simulink model "ClimaCmftStMgr"  (R2022b)
settings: Solver=FixedStepDiscrete  StartTime=0.0  StopTime=10.0  FixedStep=0.1  RelTol=1e-3
(part 1 of 2)
settings Model: Creator=blue.ge  LastModifiedBy=blue.ge
settings DataIOCC: SaveFormat=StructureWithTime
settings OptimizationCC: DefaultParameterBehavior=Inlined  EnhancedBackFolding=on  PassReuseOutputArgsAs=Individual arguments  ZeroExternalMemoryAtStartup=off  ZeroInternalMemoryAtStartup=off  OptimizeBlockOrder=speed  DifferentSizesBufferReuse=on  OptimizationCustomize=off  EfficientTunableParamExpr=on
settings DebuggingCC: ParameterTunabilityLossMsg=error
settings ModelReferenceCC: ModelReferenceNumInstancesAllowed=Single
settings SFSimCC: MATLABDynamicMemAlloc=off
settings RTWCC: Description=AUTOSAR  SystemTargetFile=autosar.tlc  GenCodeOnly=on  TemplateMakefile=ert_default_tmf
settings CodeAppCC: ForceParamTrailComments=on  IgnoreCustomStorageClasses=off  OperatorAnnotations=on  SimulinkDataObjDesc=on  SFDataObjDesc=on  InsertBlockDesc=on
settings STFCustomTargetCC: TargetLangStandard=C99 (ISO)  MultiwordLength=256  ParMdlRefBuildCompliant=on  CompOptLevelCompliant=on  IncludeMdlTerminateFcn=off  CombineOutputUpdateFcns=on  SuppressErrorStatus=on  ERTCustomFileBanners=on  SupportNonFinite=off  SupportComplex=off  SupportContinuousTime=off  SupportNonInlinedSFcns=off  RemoveResetFunc=on  MATLABClassNameForMDSCustomization=Simulink.SoftwareTarget.ERTCustomization  AutosarCompliant=on  UseToolchainInfoCompliant=on  LookupTableObjectStructAxisOrder=2,1,3,4,...  LUTObjectStructOrderEvenSpacing=Size,Table,Breakpoints  SystemTargetFile=autosar.tlc
settings ERTTargetCC: TargetLangStandard=C99 (ISO)  ConcurrentExecutionCompliant=off  IncludeMdlTerminateFcn=off  SuppressErrorStatus=on  SupportNonFinite=off  SupportComplex=off  ModelStepFunctionPrototypeControlCompliant=off  CPPClassGenCompliant=off  AutosarCompliant=on  LookupTableObjectStructAxisOrder=2,1,3,4,...  LUTObjectStructOrderEvenSpacing=Size,Table,Breakpoints  InlinedParameterPlacement=NonHierarchical  ERTCustomFileTemplate=example_file_process.tlc

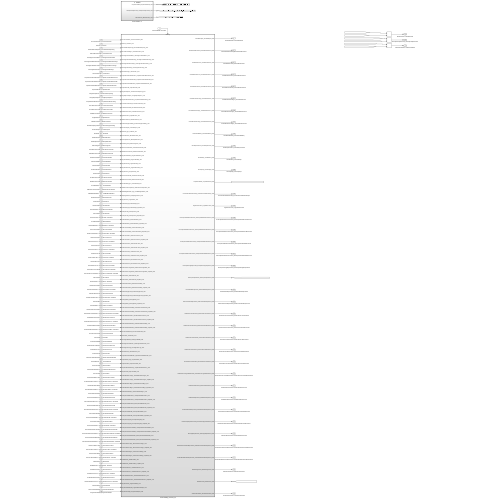
[thumbnail: Simulink's stored preview of the top-level diagram]
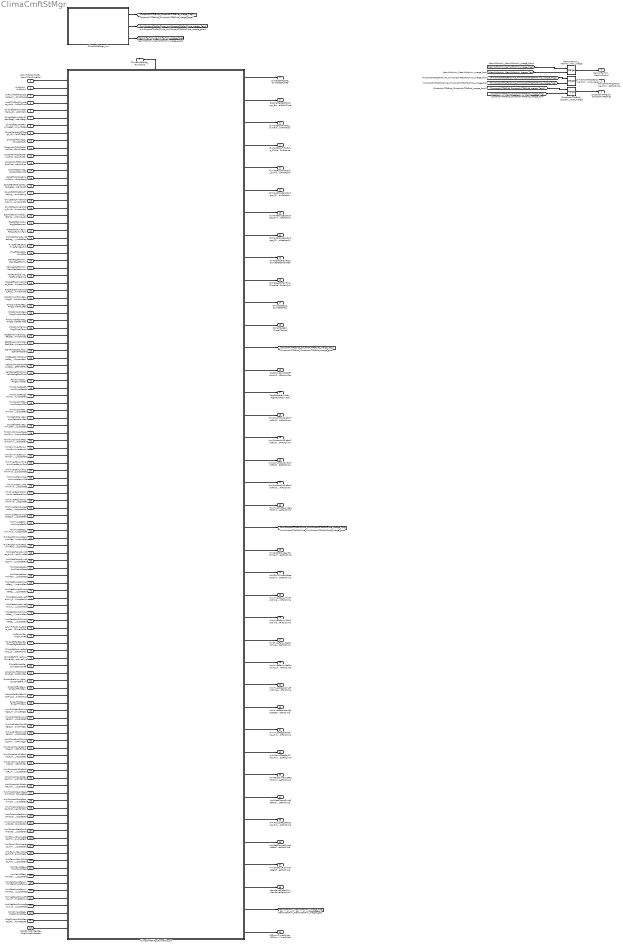
== system ClimaCmftStMgr ==
  ClimaCmftStMgr_Runnable : Inport  SampleTime=0.1  OutputFunctionCall=on
  ActvnTiForClimaCtrl_ActvnTiForClimaCtrl : Inport  Port=2  OutDataTypeStr=Ti33  PortDimensions=1
  AirCdnAvl_AirCdnAvl : Inport  Port=3  OutDataTypeStr=Boolean  PortDimensions=1
  AirFlowAddlForDispCoolg_AirFlowAddlForDispCoolg : Inport  Port=4  OutDataTypeStr=AirFlow30  PortDimensions=1
  AmbTFildForClimaNgcc_AmbTFildForClima : Inport  Port=5  OutDataTypeStr=T30  PortDimensions=1
  ClimaCfgForClimaCtrlMan1_ClimaCfgForClimaCtrlMan1 : Inport  Port=6  OutDataTypeStr=Boolean  PortDimensions=1
  ClimaCfgForNrOfAirDistbnSetg1_ClimaCfgForNrOfAirDistbnSetg1 : Inport  Port=7  OutDataTypeStr=Enum: ClimaCfgForNrOfAirDistbnSetg1  PortDimensions=1
  ClimaCfgForNrOfFanLvlSetg1_ClimaCfgForNrOfFanLvlSetg1 : Inport  Port=8  OutDataTypeStr=Enum: ClimaCfgForNrOfFanLvlSetg1  PortDimensions=1
  ClimaCfgForNrOfTSetg1_ClimaCfgForNrOfTSetg1 : Inport  Port=9  OutDataTypeStr=Enum: ClimaCfgForNrOfTSetg1  PortDimensions=1
  ClimaCtrlActvNgcc_ClimaCtrlActv : Inport  Port=10  OutDataTypeStr=Boolean  PortDimensions=1
  CmptmtAirTCtrlErrAtRowFirstLe_CmptmtAirTCtrlErrAtRowFirstLe : Inport  Port=11  OutDataTypeStr=T30  PortDimensions=1
  CmptmtAirTCtrlErrAtRowFirstRi_CmptmtAirTCtrlErrAtRowFirstRi : Inport  Port=12  OutDataTypeStr=T30  PortDimensions=1
  CmptmtAirTEstimdAtRowFirst_CmptmtAirTEstimdAtRowFirst : Inport  Port=13  OutDataTypeStr=T30  PortDimensions=1
  CmptmtObsvrSts_CmptmtObsvrSts : Inport  Port=14  OutDataTypeStr=Enum: ObsvrStsForCmptmt  PortDimensions=1
  CoolgForDispActvd_AirCdnActvdForDispCoolg : Inport  Port=15  OutDataTypeStr=Boolean  PortDimensions=1
  CoolgReqForDispActv_CoolgReqForDispActv : Inport  Port=16  OutDataTypeStr=Enum: CoolgReqForDisp  PortDimensions=1
  CoupldCtrlErrAtRowFirstAvg_CoupldCtrlErrAtRowFirstAvg : Inport  Port=17  OutDataTypeStr=T30  PortDimensions=1
  DrvrLeElecAirVentnSts_DrvrLeElecAirVentnSts : Inport  Port=18  OutDataTypeStr=Enum: OnOff1  PortDimensions=1
  DrvrRiElecAirVentnSts_DrvrRiElecAirVentnSts : Inport  Port=19  OutDataTypeStr=Enum: OnOff1  PortDimensions=1
  EcoModForClimaActv_EcoModForClimaActv : Inport  Port=20  OutDataTypeStr=Enum: OnOff1  PortDimensions=1
  EngStrtRemActv_EngStrtRemActv : Inport  Port=21  OutDataTypeStr=Boolean  PortDimensions=1
  FltModForHmiFailr_FltModForHmiFailr : Inport  Port=22  OutDataTypeStr=Boolean  PortDimensions=1
  HmiPassTempSyncSetFlag_HmiPassTempSyncSetFlag : Inport  Port=23  OutDataTypeStr=Boolean  PortDimensions=1
  HvacFanReqFrnt_HvacFanReqFrnt : Inport  Port=24  OutDataTypeStr=HvacFanSpd  PortDimensions=1
  IHUAPSts_Ngcc_IHUAPSts : Inport  Port=25  OutDataTypeStr=Enum: OnOff1  PortDimensions=1
  MemReadForHmi_MemReadForHmi : Inport  Port=26  OutDataTypeStr=Bus: MemRecForHmi  PortDimensions=1  BusOutputAsStruct=on
  MemReqStsForHmi_MemReqStsForHmi : Inport  Port=27  OutDataTypeStr=Enum: MemReqSts  PortDimensions=1
  ModForPrkgClima_ModForPrkgClima : Inport  Port=28  OutDataTypeStr=Enum: PrkgClimaMod  PortDimensions=1
  PassLeElecAirVentnSts_PassLeElecAirVentnSts : Inport  Port=29  OutDataTypeStr=Enum: OnOff1  PortDimensions=1
  PassRiElecAirVentnSts_PassRiElecAirVentnSts : Inport  Port=30  OutDataTypeStr=Enum: OnOff1  PortDimensions=1
  PrkgClimaAirflowReq_PrkgClimaAirflowReq : Inport  Port=31  OutDataTypeStr=Enum: HmiHvacFanLvl  PortDimensions=1
  PrkgClimaModReq_PrkgClimaModReq : Inport  Port=32  OutDataTypeStr=Enum: HmiCmptmtAirDistbnFrnt  PortDimensions=1
  PrkgClimaOnReq_PrkgClimaOnReq : Inport  Port=33  OutDataTypeStr=Boolean  PortDimensions=1
  PrkgClimaRecirReq_PrkgClimaRecirReq : Inport  Port=34  OutDataTypeStr=Enum: HmiHvacRecircCmd  PortDimensions=1
  PrkgClimaTemp_PrkgClimaTemp : Inport  Port=35  OutDataTypeStr=T30  PortDimensions=1
  ReLeElecAirVentnSts_ReLeElecAirVentnSts : Inport  Port=36  OutDataTypeStr=Enum: OnOff1  PortDimensions=1
  ReRiElecAirVentnSts_ReRiElecAirVentnSts : Inport  Port=37  OutDataTypeStr=Enum: OnOff1  PortDimensions=1
  SceneModSeld_Ngcc_SceneModSeld : Inport  Port=38  OutDataTypeStr=Enum: PatSeld  PortDimensions=1
  TiOfDeactvnForCmptmtFan_TiOfDeactvnForCmptmtFan : Inport  Port=39  OutDataTypeStr=Ti33  PortDimensions=1
  VehModMngtGlbSafe1_Ngcc_VehModMngtGlbSafe1 : Inport  Port=40  OutDataTypeStr=Bus: VehModMngtGlbSafe1  PortDimensions=1  BusOutputAsStruct=on
  VehSpdLgtForClima_VehSpdLgtForClima : Inport  Port=41  OutDataTypeStr=Bus: VehSpdLgtForClima  PortDimensions=1  BusOutputAsStruct=on
  PrkgClimaActv_PrkgClimaActv : Inport  Port=42  OutDataTypeStr=Enum: PrkgClimaActv  PortDimensions=1
  HmiClimaReqQf_HmiClimaReqQf : Inport  Port=43  OutDataTypeStr=Enum: HmiClimaReqQf  PortDimensions=1
  HmiClimaReqQf_HmiClimaReqQf_IsUpdated : Inport  Port=44  OutDataTypeStr=boolean  PortDimensions=1
  HmiCmptmtTSp_HmiCmptmtTSp : Inport  Port=45  OutDataTypeStr=Bus: HmiCmptmtTSp  PortDimensions=1  BusOutputAsStruct=on
  HmiCmptmtTSp_HmiCmptmtTSp_IsUpdated : Inport  Port=46  OutDataTypeStr=boolean  PortDimensions=1
  HmiDefrstMaxReq_HmiDefrstMaxReq : Inport  Port=47  OutDataTypeStr=Enum: ActrReq  PortDimensions=1
  HmiDefrstMaxReq_HmiDefrstMaxReq_IsUpdated : Inport  Port=48  OutDataTypeStr=boolean  PortDimensions=1
  HmiDrvrClimaAutReq_HmiDrvrClimaAutReq : Inport  Port=49  OutDataTypeStr=Enum: OnOff1  PortDimensions=1
  HmiDrvrClimaAutReq_HmiDrvrClimaAutReq_IsUpdated : Inport  Port=50  OutDataTypeStr=boolean  PortDimensions=1
  HmiDrvrHvacFanLvl_HmiDrvrHvacFanLvl : Inport  Port=51  OutDataTypeStr=Enum: HmiHvacFanLvl  PortDimensions=1
  HmiDrvrHvacFanLvl_HmiDrvrHvacFanLvl_IsUpdated : Inport  Port=52  OutDataTypeStr=boolean  PortDimensions=1
  HmiHvacFanLvlFrnt_HmiHvacFanLvlFrnt : Inport  Port=53  OutDataTypeStr=Enum: HmiHvacFanLvl  PortDimensions=1
  HmiHvacFanLvlFrnt_HmiHvacFanLvlFrnt_IsUpdated : Inport  Port=54  OutDataTypeStr=boolean  PortDimensions=1
  HmiHvacFanLvlRe_HmiHvacFanLvlRe : Inport  Port=55  OutDataTypeStr=Enum: HmiHvacFanLvl  PortDimensions=1
  HmiHvacFanLvlRe_HmiHvacFanLvlRe_IsUpdated : Inport  Port=56  OutDataTypeStr=boolean  PortDimensions=1
  HmiHvacRecircCmd_HmiHvacRecircCmd : Inport  Port=57  OutDataTypeStr=Enum: HmiHvacRecircCmd  PortDimensions=1
  HmiHvacRecircCmd_HmiHvacRecircCmd_IsUpdated : Inport  Port=58  OutDataTypeStr=boolean  PortDimensions=1
  HmiHvacRecircLongPsdSts_HmiHvacRecircLongPsdSts : Inport  Port=59  OutDataTypeStr=Enum: OnOff1  PortDimensions=1
  HmiHvacRecircLongPsdSts_HmiHvacRecircLongPsdSts_IsUpdated : Inport  Port=60  OutDataTypeStr=boolean  PortDimensions=1
  HmiHvacReCtrl_HmiHvacReCtrl : Inport  Port=61  OutDataTypeStr=Enum: HmiHvacReCtrl  PortDimensions=1
  HmiHvacReCtrl_HmiHvacReCtrl_IsUpdated : Inport  Port=62  OutDataTypeStr=boolean  PortDimensions=1
  HmiPassClimaAutReq_HmiPassClimaAutReq : Inport  Port=63  OutDataTypeStr=Enum: OnOff1  PortDimensions=1
  HmiPassClimaAutReq_HmiPassClimaAutReq_IsUpdated : Inport  Port=64  OutDataTypeStr=boolean  PortDimensions=1
  HmiPassTempSyncSet_HmiPassTempSyncSet : Inport  Port=65  OutDataTypeStr=Enum: OnOff1  PortDimensions=1
  HmiPassTempSyncSet_HmiPassTempSyncSet_IsUpdated : Inport  Port=66  OutDataTypeStr=boolean  PortDimensions=1
  HmiPopUpResp_HmiPopUpResp : Inport  Port=67  OutDataTypeStr=Enum: BookChargeSetResponse  PortDimensions=1
  HmiPopUpResp_HmiPopUpResp_IsUpdated : Inport  Port=68  OutDataTypeStr=boolean  PortDimensions=1
  HmiSecRowLeClimaAutReq_HmiSecRowLeClimaAutReq : Inport  Port=69  OutDataTypeStr=Enum: OnOff1  PortDimensions=1
  HmiSecRowLeClimaAutReq_HmiSecRowLeClimaAutReq_IsUpdated : Inport  Port=70  OutDataTypeStr=boolean  PortDimensions=1
  HmiSecRowLeHvacFanLvl_HmiSecRowLeHvacFanLvl : Inport  Port=71  OutDataTypeStr=Enum: HmiHvacFanLvl  PortDimensions=1
  HmiSecRowLeHvacFanLvl_HmiSecRowLeHvacFanLvl_IsUpdated : Inport  Port=72  OutDataTypeStr=boolean  PortDimensions=1
  HmiSecRowRiClimaAutReq_HmiSecRowRiClimaAutReq : Inport  Port=73  OutDataTypeStr=Enum: OnOff1  PortDimensions=1
  HmiSecRowRiClimaAutReq_HmiSecRowRiClimaAutReq_IsUpdated : Inport  Port=74  OutDataTypeStr=boolean  PortDimensions=1
  ActvnTiForClimaCtrlRe_ActvnTiForClimaCtrlRe : Inport  Port=75  OutDataTypeStr=Ti33  PortDimensions=1
  AirCdnAvlRe_AirCdnAvlRe : Inport  Port=76  OutDataTypeStr=Boolean  PortDimensions=1
  ClimaCfgForBRAND_ClimaCfgForBRAND : Inport  Port=77  OutDataTypeStr=Enum: ClimaCfgForBRAND  PortDimensions=1
  ClimaCfgForHvacRePrsnt1_ClimaCfgForHvacRePrsnt1 : Inport  Port=78  OutDataTypeStr=Enum: CarCfgForHvacRe  PortDimensions=1
  ClimaCfgForHvacTyp_ClimaCfgForHvacTyp : Inport  Port=79  OutDataTypeStr=Enum: CarCfgForHvacTyp2  PortDimensions=1
  ClimaCtrlActvRe_ClimaCtrlActvRe : Inport  Port=80  OutDataTypeStr=Boolean  PortDimensions=1
  CmptmtAirTEstimdAtRowSec_CmptmtAirTEstimdAtRowSec : Inport  Port=81  OutDataTypeStr=T30  PortDimensions=1
  CmptmtRelHum_Ngcc_CmptmtRelHum : Inport  Port=82  OutDataTypeStr=Bus: CmptmRelHum  PortDimensions=1  BusOutputAsStruct=on
  CmptmtTFrntRaw_CmptmtTFrntRaw : Inport  Port=83  OutDataTypeStr=Bus: CmptmtTFrnt  PortDimensions=1  BusOutputAsStruct=on
  CoupldCtrlErrAtRowSecAvg_CoupldCtrlErrAtRowSecAvg : Inport  Port=84  OutDataTypeStr=T30  PortDimensions=1
  EvaprTFrntRaw_EvaprTFrntRaw : Inport  Port=85  OutDataTypeStr=Bus: EvaprTFrnt  PortDimensions=1  BusOutputAsStruct=on
  HmiAirDistbnRow1LeNgcc_HmiAirDistbnRow1LeNgcc : Inport  Port=86  OutDataTypeStr=Enum: HmiCmptmtAirDistbnFrnt  PortDimensions=1
  HmiAirDistbnRow1LeNgcc_HmiAirDistbnRow1LeNgcc_IsUpdated : Inport  Port=87  OutDataTypeStr=boolean  PortDimensions=1
  HmiAirDistbnRow1RiNgcc_HmiAirDistbnRow1RiNgcc : Inport  Port=88  OutDataTypeStr=Enum: HmiCmptmtAirDistbnFrnt  PortDimensions=1
  HmiAirDistbnRow1RiNgcc_HmiAirDistbnRow1RiNgcc_IsUpdated : Inport  Port=89  OutDataTypeStr=boolean  PortDimensions=1
  HmiClimaRow3PwrNgcc_HmiClimaRow3PwrNgcc : Inport  Port=90  OutDataTypeStr=Enum: OnOff1  PortDimensions=1
  HmiCmptmtAirDistbnFrntLe_HmiCmptmtAirDistbnFrntLe : Inport  Port=91  OutDataTypeStr=Enum: HmiCmptmtAirDistbnFrnt  PortDimensions=1
  HmiCmptmtAirDistbnFrntLe_HmiCmptmtAirDistbnFrntLe_IsUpdated : Inport  Port=92  OutDataTypeStr=boolean  PortDimensions=1
  HmiCmptmtAirDistbnFrntRi_HmiCmptmtAirDistbnFrntRi : Inport  Port=93  OutDataTypeStr=Enum: HmiCmptmtAirDistbnFrnt  PortDimensions=1
  HmiCmptmtAirDistbnFrntRi_HmiCmptmtAirDistbnFrntRi_IsUpdated : Inport  Port=94  OutDataTypeStr=boolean  PortDimensions=1
  HmiCmptmtAirDistbnRe_HmiCmptmtAirDistbnRe : Inport  Port=95  OutDataTypeStr=Enum: HmiCmptmtAirDistbnFrnt  PortDimensions=1
  HmiCmptmtAirDistbnRe_HmiCmptmtAirDistbnRe_IsUpdated : Inport  Port=96  OutDataTypeStr=boolean  PortDimensions=1
  HmiCmptmtCoolgReq_HmiCmptmtCoolgReq : Inport  Port=97  OutDataTypeStr=Enum: HmiCmptmtCoolgReq  PortDimensions=1
  HmiCmptmtCoolgReq_HmiCmptmtCoolgReq_IsUpdated : Inport  Port=98  OutDataTypeStr=boolean  PortDimensions=1
  HmiCmptmtSecRowLeAirDistbn_HmiCmptmtSecRowLeAirDistbn : Inport  Port=99  OutDataTypeStr=Enum: HmiCmptmtAirDistbnFrnt  PortDimensions=1
  HmiCmptmtSecRowLeAirDistbn_HmiCmptmtSecRowLeAirDistbn_IsUpdated : Inport  Port=100  OutDataTypeStr=boolean  PortDimensions=1
  HmiCmptmtSecRowRiAirDistbn_HmiCmptmtSecRowRiAirDistbn : Inport  Port=101  OutDataTypeStr=Enum: HmiCmptmtAirDistbnFrnt  PortDimensions=1
  HmiCmptmtSecRowRiAirDistbn_HmiCmptmtSecRowRiAirDistbn_IsUpdated : Inport  Port=102  OutDataTypeStr=boolean  PortDimensions=1
  HmiFanLvlRow1LeNgcc_HmiFanLvlRow1LeNgcc : Inport  Port=103  OutDataTypeStr=Enum: HmiHvacFanLvl  PortDimensions=1
  HmiFanLvlRow1LeNgcc_HmiFanLvlRow1LeNgcc_IsUpdated : Inport  Port=104  OutDataTypeStr=boolean  PortDimensions=1
  HmiFanLvlRow1RiNgcc_HmiFanLvlRow1RiNgcc : Inport  Port=105  OutDataTypeStr=Enum: HmiHvacFanLvl  PortDimensions=1
  HmiFanLvlRow1RiNgcc_HmiFanLvlRow1RiNgcc_IsUpdated : Inport  Port=106  OutDataTypeStr=boolean  PortDimensions=1
  HmiMaxACReq_HmiMaxACReq : Inport  Port=107  OutDataTypeStr=Enum: OnOff1  PortDimensions=1
  HmiMaxACReq_HmiMaxACReq_IsUpdated : Inport  Port=108  OutDataTypeStr=boolean  PortDimensions=1
  HmiPassHvacFanLvl_HmiPassHvacFanLvl : Inport  Port=109  OutDataTypeStr=Enum: HmiHvacFanLvl  PortDimensions=1
  HmiPassHvacFanLvl_HmiPassHvacFanLvl_IsUpdated : Inport  Port=110  OutDataTypeStr=boolean  PortDimensions=1
  HmiSecRowRiHvacFanLvl_HmiSecRowRiHvacFanLvl : Inport  Port=111  OutDataTypeStr=Enum: HmiHvacFanLvl  PortDimensions=1
  HmiSecRowRiHvacFanLvl_HmiSecRowRiHvacFanLvl_IsUpdated : Inport  Port=112  OutDataTypeStr=boolean  PortDimensions=1
  PrkgClimaACReq_PrkgClimaACReq : Inport  Port=113  OutDataTypeStr=Enum: HmiCmptmtCoolgReq  PortDimensions=1
  PrkgClimaAirflowReqRe_PrkgClimaAirflowReqRe : Inport  Port=114  OutDataTypeStr=Enum: HmiHvacFanLvl  PortDimensions=1
  PrkgClimaOnReqRe_PrkgClimaOnReqRe : Inport  Port=115  OutDataTypeStr=Enum: OnOff1  PortDimensions=1
  ClimaCmftStMgr_Init : Subsystem
  ClimaCmftStMgr_Runnable_sys : Subsystem
  CmptmtAirTFbExtd_CmptmtAirTFbExtd_merge : Merge
  HmiCmptmtTSpForClima_HmiCmptmtTSpForClima_merge : Merge
  MemWrForHmi_MemWrForHmi_merge : Merge
  CmptmtAirTFbExtd_CmptmtAirTFbExtd : Outport  OutDataTypeStr=Bus: CmptmtAirTEstimdExtd  PortDimensions=1  BusOutputAsStruct=on
  HmiCmptmtTSpForClima_HmiCmptmtTSpForClima : Outport  Port=2  OutDataTypeStr=Bus: HmiCmptmtTSp  PortDimensions=1  BusOutputAsStruct=on
  MemWrForHmi_MemWrForHmi : Outport  Port=3  OutDataTypeStr=Bus: MemRecForHmi  PortDimensions=1  BusOutputAsStruct=on
  ClimaStStrtUpRe_ClimaStStrtUpRe : Outport  Port=4  OutDataTypeStr=Enum: ClimaStStrtUp  PortDimensions=1
  BiochalPatseldForClima_BiochalPatseldForClima : Outport  Port=5  OutDataTypeStr=Boolean  PortDimensions=1
  ClimaAtRowFrntActv_ClimaAtRowFrntActv : Outport  Port=6  OutDataTypeStr=Boolean  PortDimensions=1
  ClimaAtRowFrntActvLe_ClimaAtRowFrntActvLe : Outport  Port=7  OutDataTypeStr=Boolean  PortDimensions=1
  ClimaAtRowFrntActvRi_ClimaAtRowFrntActvRi : Outport  Port=8  OutDataTypeStr=Boolean  PortDimensions=1
  ClimaAtRowSecActvNgcc_ClimaAtRowSecActv : Outport  Port=9  OutDataTypeStr=Boolean  PortDimensions=1
  ClimaAtRowSecActvNgcc_ClimaAtRowSecActvLe : Outport  Port=10  OutDataTypeStr=Boolean  PortDimensions=1
  ClimaAtRowSecActvNgcc_ClimaAtRowSecActvRi : Outport  Port=11  OutDataTypeStr=Boolean  PortDimensions=1
  ClimaDefrstMaxReq_ClimaDefrstMaxReq : Outport  Port=12  OutDataTypeStr=Boolean  PortDimensions=1
  ClimaReqForFanFrnt_ClimaReqForFanFrnt : Outport  Port=13  OutDataTypeStr=Boolean  PortDimensions=1
  ClimaStStrtUp_ClimaStStrtUp : Outport  Port=14  OutDataTypeStr=Enum: ClimaStStrtUp  PortDimensions=1
  ClimaTSpSpcl_ClimaTSpSpcl : Outport  Port=15  OutDataTypeStr=Bus: ClimaTSpSpcl  PortDimensions=1  BusOutputAsStruct=on
  CustClimaOvrdNotifForHmiCpt_CustClimaOvrdNotifForHmiCpt : Outport  Port=16  OutDataTypeStr=Enum: Flg1  PortDimensions=1
  FogFlashPrevnActv_FogFlashPrevnActv : Outport  Port=17  OutDataTypeStr=Boolean  PortDimensions=1
  HmiCmptmtAirDistbnFrntForClima_HmiCmptmtAirDistbnFrntForClima : Outport  Port=18  OutDataTypeStr=Enum: HmiCmptmtAirDistbnFrnt  PortDimensions=1
  HmiCmptmtAirDistbnFrntForClima_HmiCmptmtAirDistbnFrntRiForClima : Outport  Port=19  OutDataTypeStr=Enum: HmiCmptmtAirDistbnFrnt  PortDimensions=1
  HmiCmptmtAirDistbnFrntForClima_HmiCmptmtAirDistbnReForClima : Outport  Port=20  OutDataTypeStr=Enum: HmiCmptmtAirDistbnFrnt  PortDimensions=1
  HmiCmptmtAirDistbnFrntForClima_HmiCmptmtAirDistbnReRiForClima : Outport  Port=21  OutDataTypeStr=Enum: HmiCmptmtAirDistbnFrnt  PortDimensions=1
  HmiCmptmtCoolgReqForClima_HmiCmptmtCoolgReqForClima : Outport  Port=22  OutDataTypeStr=Enum: HmiCmptmtCoolgReq  PortDimensions=1
  HmiDefrstMaxReqForClima_HmiDefrstMaxReqForClima : Outport  Port=23  OutDataTypeStr=Enum: ActrReq  PortDimensions=1
  HmiDrvrClimaAutReqForClima_HmiDrvrClimaAutReqForClima : Outport  Port=24  OutDataTypeStr=Enum: OnOff1  PortDimensions=1
  HmiHvacFanLvlFrntForClima_HmiHvacFanLvlFrntForClima : Outport  Port=25  OutDataTypeStr=Enum: HmiHvacFanLvl  PortDimensions=1
  HmiHvacFanLvlFrntForClima_HmiHvacFanLvlFrntRiForClima : Outport  Port=26  OutDataTypeStr=Enum: HmiHvacFanLvl  PortDimensions=1
  HmiHvacFanLvlReForClima_HmiHvacFanLvlReForClima : Outport  Port=27  OutDataTypeStr=Enum: HmiHvacFanLvl  PortDimensions=1
  HmiHvacFanLvlReForClima_HmiHvacFanLvlReRiForClima : Outport  Port=28  OutDataTypeStr=Enum: HmiHvacFanLvl  PortDimensions=1
  HmiHvacRecircCmdForClima_HmiHvacRecircCmdForClima : Outport  Port=29  OutDataTypeStr=Enum: HmiHvacRecircCmd  PortDimensions=1
  HmiHvacRecircLongPsdStsForClima_HmiHvacRecircLongPsdStsForClima : Outport  Port=30  OutDataTypeStr=Enum: OnOff1  PortDimensions=1
  HmiHvacReCtrlForClima_HmiHvacReCtrlForClima : Outport  Port=31  OutDataTypeStr=Enum: HmiHvacReCtrl  PortDimensions=1
  HmiMaxACReqForClima_HmiMaxACReqForClima : Outport  Port=32  OutDataTypeStr=Enum: OnOff1  PortDimensions=1
  HmiPassClimaAutReqForClima_HmiPassClimaAutReqForClima : Outport  Port=33  OutDataTypeStr=Enum: OnOff1  PortDimensions=1
  HmiPassTempSyncSetForClima_HmiPassTempSyncSetForClima : Outport  Port=34  OutDataTypeStr=Enum: OnOff1  PortDimensions=1
  HmiPopUpRespForClima_HmiPopUpRespForClima : Outport  Port=35  OutDataTypeStr=Enum: BookChargeSetResponse  PortDimensions=1
  HmiSecRowLeClimaAutReqForClima_HmiSecRowLeClimaAutReqForClima : Outport  Port=36  OutDataTypeStr=Enum: OnOff1  PortDimensions=1
  HmiSecRowRiClimaAutReqForClima_HmiSecRowRiClimaAutReqForClima : Outport  Port=37  OutDataTypeStr=Enum: OnOff1  PortDimensions=1
  MemSaveFlgForHmi_MemSaveFlgForHmi : Outport  Port=38  OutDataTypeStr=Boolean  PortDimensions=1
  VFCHmiClimaCtrlAct_VFCHmiClimaCtrlAct : Outport  Port=39  OutDataTypeStr=Enum: OnOff1  PortDimensions=1
  wires:
  ActvnTiForClimaCtrl_ActvnTiForClimaCtrl/1 -> ClimaCmftStMgr_Runnable_sys/1(ActvnTiForClimaCtrl_ActvnTiForClimaCtrl_read)
  AirCdnAvl_AirCdnAvl/1 -> ClimaCmftStMgr_Runnable_sys/2(AirCdnAvl_AirCdnAvl_read)
  AirFlowAddlForDispCoolg_AirFlowAddlForDispCoolg/1 -> ClimaCmftStMgr_Runnable_sys/3(AirFlowAddlForDispCoolg_AirFlowAddlForDispCoolg_read)
  AmbTFildForClimaNgcc_AmbTFildForClima/1 -> ClimaCmftStMgr_Runnable_sys/4(AmbTFildForClimaNgcc_AmbTFildForClima_read)
  ClimaCfgForClimaCtrlMan1_ClimaCfgForClimaCtrlMan1/1 -> ClimaCmftStMgr_Runnable_sys/5(ClimaCfgForClimaCtrlMan1_ClimaCfgForClimaCtrlMan1_read)
  ClimaCfgForNrOfAirDistbnSetg1_ClimaCfgForNrOfAirDistbnSetg1/1 -> ClimaCmftStMgr_Runnable_sys/6(ClimaCfgForNrOfAirDistbnSetg1_ClimaCfgForNrOfAirDistbnSetg1_read)
  ClimaCfgForNrOfFanLvlSetg1_ClimaCfgForNrOfFanLvlSetg1/1 -> ClimaCmftStMgr_Runnable_sys/7(ClimaCfgForNrOfFanLvlSetg1_ClimaCfgForNrOfFanLvlSetg1_read)
  ClimaCfgForNrOfTSetg1_ClimaCfgForNrOfTSetg1/1 -> ClimaCmftStMgr_Runnable_sys/8(ClimaCfgForNrOfTSetg1_ClimaCfgForNrOfTSetg1_read)
  ClimaCtrlActvNgcc_ClimaCtrlActv/1 -> ClimaCmftStMgr_Runnable_sys/9(ClimaCtrlActvNgcc_ClimaCtrlActv_read)
  CmptmtAirTCtrlErrAtRowFirstLe_CmptmtAirTCtrlErrAtRowFirstLe/1 -> ClimaCmftStMgr_Runnable_sys/10(CmptmtAirTCtrlErrAtRowFirstLe_CmptmtAirTCtrlErrAtRowFirstLe_read)
  CmptmtAirTCtrlErrAtRowFirstRi_CmptmtAirTCtrlErrAtRowFirstRi/1 -> ClimaCmftStMgr_Runnable_sys/11(CmptmtAirTCtrlErrAtRowFirstRi_CmptmtAirTCtrlErrAtRowFirstRi_read)
  CmptmtAirTEstimdAtRowFirst_CmptmtAirTEstimdAtRowFirst/1 -> ClimaCmftStMgr_Runnable_sys/12(CmptmtAirTEstimdAtRowFirst_CmptmtAirTEstimdAtRowFirst_read)
  CmptmtObsvrSts_CmptmtObsvrSts/1 -> ClimaCmftStMgr_Runnable_sys/13(CmptmtObsvrSts_CmptmtObsvrSts_read)
  CoolgForDispActvd_AirCdnActvdForDispCoolg/1 -> ClimaCmftStMgr_Runnable_sys/14(CoolgForDispActvd_AirCdnActvdForDispCoolg_read)
  CoolgReqForDispActv_CoolgReqForDispActv/1 -> ClimaCmftStMgr_Runnable_sys/15(CoolgReqForDispActv_CoolgReqForDispActv_read)
  CoupldCtrlErrAtRowFirstAvg_CoupldCtrlErrAtRowFirstAvg/1 -> ClimaCmftStMgr_Runnable_sys/16(CoupldCtrlErrAtRowFirstAvg_CoupldCtrlErrAtRowFirstAvg_read)
  DrvrLeElecAirVentnSts_DrvrLeElecAirVentnSts/1 -> ClimaCmftStMgr_Runnable_sys/17(DrvrLeElecAirVentnSts_DrvrLeElecAirVentnSts_read)
  DrvrRiElecAirVentnSts_DrvrRiElecAirVentnSts/1 -> ClimaCmftStMgr_Runnable_sys/18(DrvrRiElecAirVentnSts_DrvrRiElecAirVentnSts_read)
  EcoModForClimaActv_EcoModForClimaActv/1 -> ClimaCmftStMgr_Runnable_sys/19(EcoModForClimaActv_EcoModForClimaActv_read)
  EngStrtRemActv_EngStrtRemActv/1 -> ClimaCmftStMgr_Runnable_sys/20(EngStrtRemActv_EngStrtRemActv_read)
  FltModForHmiFailr_FltModForHmiFailr/1 -> ClimaCmftStMgr_Runnable_sys/21(FltModForHmiFailr_FltModForHmiFailr_read)
  HmiPassTempSyncSetFlag_HmiPassTempSyncSetFlag/1 -> ClimaCmftStMgr_Runnable_sys/22(HmiPassTempSyncSetFlag_HmiPassTempSyncSetFlag_read)
  HvacFanReqFrnt_HvacFanReqFrnt/1 -> ClimaCmftStMgr_Runnable_sys/23(HvacFanReqFrnt_HvacFanReqFrnt_read)
  IHUAPSts_Ngcc_IHUAPSts/1 -> ClimaCmftStMgr_Runnable_sys/24(IHUAPSts_Ngcc_IHUAPSts_read)
  MemReadForHmi_MemReadForHmi/1 -> ClimaCmftStMgr_Runnable_sys/25(MemReadForHmi_MemReadForHmi_read)
  MemReqStsForHmi_MemReqStsForHmi/1 -> ClimaCmftStMgr_Runnable_sys/26(MemReqStsForHmi_MemReqStsForHmi_read)
  ModForPrkgClima_ModForPrkgClima/1 -> ClimaCmftStMgr_Runnable_sys/27(ModForPrkgClima_ModForPrkgClima_read)
  PassLeElecAirVentnSts_PassLeElecAirVentnSts/1 -> ClimaCmftStMgr_Runnable_sys/28(PassLeElecAirVentnSts_PassLeElecAirVentnSts_read)
  PassRiElecAirVentnSts_PassRiElecAirVentnSts/1 -> ClimaCmftStMgr_Runnable_sys/29(PassRiElecAirVentnSts_PassRiElecAirVentnSts_read)
  PrkgClimaAirflowReq_PrkgClimaAirflowReq/1 -> ClimaCmftStMgr_Runnable_sys/30(PrkgClimaAirflowReq_PrkgClimaAirflowReq_read)
  PrkgClimaModReq_PrkgClimaModReq/1 -> ClimaCmftStMgr_Runnable_sys/31(PrkgClimaModReq_PrkgClimaModReq_read)
  PrkgClimaOnReq_PrkgClimaOnReq/1 -> ClimaCmftStMgr_Runnable_sys/32(PrkgClimaOnReq_PrkgClimaOnReq_read)
  PrkgClimaRecirReq_PrkgClimaRecirReq/1 -> ClimaCmftStMgr_Runnable_sys/33(PrkgClimaRecirReq_PrkgClimaRecirReq_read)
  PrkgClimaTemp_PrkgClimaTemp/1 -> ClimaCmftStMgr_Runnable_sys/34(PrkgClimaTemp_PrkgClimaTemp_read)
  ReLeElecAirVentnSts_ReLeElecAirVentnSts/1 -> ClimaCmftStMgr_Runnable_sys/35(ReLeElecAirVentnSts_ReLeElecAirVentnSts_read)
  ReRiElecAirVentnSts_ReRiElecAirVentnSts/1 -> ClimaCmftStMgr_Runnable_sys/36(ReRiElecAirVentnSts_ReRiElecAirVentnSts_read)
  SceneModSeld_Ngcc_SceneModSeld/1 -> ClimaCmftStMgr_Runnable_sys/37(SceneModSeld_Ngcc_SceneModSeld_read)
  TiOfDeactvnForCmptmtFan_TiOfDeactvnForCmptmtFan/1 -> ClimaCmftStMgr_Runnable_sys/38(TiOfDeactvnForCmptmtFan_TiOfDeactvnForCmptmtFan_read)
  VehModMngtGlbSafe1_Ngcc_VehModMngtGlbSafe1/1 -> ClimaCmftStMgr_Runnable_sys/39(VehModMngtGlbSafe1_Ngcc_VehModMngtGlbSafe1_read)
  VehSpdLgtForClima_VehSpdLgtForClima/1 -> ClimaCmftStMgr_Runnable_sys/40(VehSpdLgtForClima_VehSpdLgtForClima_read)
  PrkgClimaActv_PrkgClimaActv/1 -> ClimaCmftStMgr_Runnable_sys/41(PrkgClimaActv_PrkgClimaActv_read)
  HmiClimaReqQf_HmiClimaReqQf/1 -> ClimaCmftStMgr_Runnable_sys/42(HmiClimaReqQf_HmiClimaReqQf_read)
  HmiClimaReqQf_HmiClimaReqQf_IsUpdated/1 -> ClimaCmftStMgr_Runnable_sys/43(HmiClimaReqQf_HmiClimaReqQf_IsUpdated_read)
  HmiCmptmtTSp_HmiCmptmtTSp/1 -> ClimaCmftStMgr_Runnable_sys/44(HmiCmptmtTSp_HmiCmptmtTSp_read)
  HmiCmptmtTSp_HmiCmptmtTSp_IsUpdated/1 -> ClimaCmftStMgr_Runnable_sys/45(HmiCmptmtTSp_HmiCmptmtTSp_IsUpdated_read)
  HmiDefrstMaxReq_HmiDefrstMaxReq/1 -> ClimaCmftStMgr_Runnable_sys/46(HmiDefrstMaxReq_HmiDefrstMaxReq_read)
  HmiDefrstMaxReq_HmiDefrstMaxReq_IsUpdated/1 -> ClimaCmftStMgr_Runnable_sys/47(HmiDefrstMaxReq_HmiDefrstMaxReq_IsUpdated_read)
  HmiDrvrClimaAutReq_HmiDrvrClimaAutReq/1 -> ClimaCmftStMgr_Runnable_sys/48(HmiDrvrClimaAutReq_HmiDrvrClimaAutReq_read)
  HmiDrvrClimaAutReq_HmiDrvrClimaAutReq_IsUpdated/1 -> ClimaCmftStMgr_Runnable_sys/49(HmiDrvrClimaAutReq_HmiDrvrClimaAutReq_IsUpdated_read)
  HmiDrvrHvacFanLvl_HmiDrvrHvacFanLvl/1 -> ClimaCmftStMgr_Runnable_sys/50(HmiDrvrHvacFanLvl_HmiDrvrHvacFanLvl_read)
  HmiDrvrHvacFanLvl_HmiDrvrHvacFanLvl_IsUpdated/1 -> ClimaCmftStMgr_Runnable_sys/51(HmiDrvrHvacFanLvl_HmiDrvrHvacFanLvl_IsUpdated_read)
  HmiHvacFanLvlFrnt_HmiHvacFanLvlFrnt/1 -> ClimaCmftStMgr_Runnable_sys/52(HmiHvacFanLvlFrnt_HmiHvacFanLvlFrnt_read)
  HmiHvacFanLvlFrnt_HmiHvacFanLvlFrnt_IsUpdated/1 -> ClimaCmftStMgr_Runnable_sys/53(HmiHvacFanLvlFrnt_HmiHvacFanLvlFrnt_IsUpdated_read)
  HmiHvacFanLvlRe_HmiHvacFanLvlRe/1 -> ClimaCmftStMgr_Runnable_sys/54(HmiHvacFanLvlRe_HmiHvacFanLvlRe_read)
  HmiHvacFanLvlRe_HmiHvacFanLvlRe_IsUpdated/1 -> ClimaCmftStMgr_Runnable_sys/55(HmiHvacFanLvlRe_HmiHvacFanLvlRe_IsUpdated_read)
  HmiHvacRecircCmd_HmiHvacRecircCmd/1 -> ClimaCmftStMgr_Runnable_sys/56(HmiHvacRecircCmd_HmiHvacRecircCmd_read)
  HmiHvacRecircCmd_HmiHvacRecircCmd_IsUpdated/1 -> ClimaCmftStMgr_Runnable_sys/57(HmiHvacRecircCmd_HmiHvacRecircCmd_IsUpdated_read)
  HmiHvacRecircLongPsdSts_HmiHvacRecircLongPsdSts/1 -> ClimaCmftStMgr_Runnable_sys/58(HmiHvacRecircLongPsdSts_HmiHvacRecircLongPsdSts_read)
  HmiHvacRecircLongPsdSts_HmiHvacRecircLongPsdSts_IsUpdated/1 -> ClimaCmftStMgr_Runnable_sys/59(HmiHvacRecircLongPsdSts_HmiHvacRecircLongPsdSts_IsUpdated_read)
  HmiHvacReCtrl_HmiHvacReCtrl/1 -> ClimaCmftStMgr_Runnable_sys/60(HmiHvacReCtrl_HmiHvacReCtrl_read)
  HmiHvacReCtrl_HmiHvacReCtrl_IsUpdated/1 -> ClimaCmftStMgr_Runnable_sys/61(HmiHvacReCtrl_HmiHvacReCtrl_IsUpdated_read)
  HmiPassClimaAutReq_HmiPassClimaAutReq/1 -> ClimaCmftStMgr_Runnable_sys/62(HmiPassClimaAutReq_HmiPassClimaAutReq_read)
  HmiPassClimaAutReq_HmiPassClimaAutReq_IsUpdated/1 -> ClimaCmftStMgr_Runnable_sys/63(HmiPassClimaAutReq_HmiPassClimaAutReq_IsUpdated_read)
  HmiPassTempSyncSet_HmiPassTempSyncSet/1 -> ClimaCmftStMgr_Runnable_sys/64(HmiPassTempSyncSet_HmiPassTempSyncSet_read)
  HmiPassTempSyncSet_HmiPassTempSyncSet_IsUpdated/1 -> ClimaCmftStMgr_Runnable_sys/65(HmiPassTempSyncSet_HmiPassTempSyncSet_IsUpdated_read)
  HmiPopUpResp_HmiPopUpResp/1 -> ClimaCmftStMgr_Runnable_sys/66(HmiPopUpResp_HmiPopUpResp_read)
  HmiPopUpResp_HmiPopUpResp_IsUpdated/1 -> ClimaCmftStMgr_Runnable_sys/67(HmiPopUpResp_HmiPopUpResp_IsUpdated_read)
  HmiSecRowLeClimaAutReq_HmiSecRowLeClimaAutReq/1 -> ClimaCmftStMgr_Runnable_sys/68(HmiSecRowLeClimaAutReq_HmiSecRowLeClimaAutReq_read)
  HmiSecRowLeClimaAutReq_HmiSecRowLeClimaAutReq_IsUpdated/1 -> ClimaCmftStMgr_Runnable_sys/69(HmiSecRowLeClimaAutReq_HmiSecRowLeClimaAutReq_IsUpdated_read)
  HmiSecRowLeHvacFanLvl_HmiSecRowLeHvacFanLvl/1 -> ClimaCmftStMgr_Runnable_sys/70(HmiSecRowLeHvacFanLvl_HmiSecRowLeHvacFanLvl_read)
  HmiSecRowLeHvacFanLvl_HmiSecRowLeHvacFanLvl_IsUpdated/1 -> ClimaCmftStMgr_Runnable_sys/71(HmiSecRowLeHvacFanLvl_HmiSecRowLeHvacFanLvl_IsUpdated_read)
  HmiSecRowRiClimaAutReq_HmiSecRowRiClimaAutReq/1 -> ClimaCmftStMgr_Runnable_sys/72(HmiSecRowRiClimaAutReq_HmiSecRowRiClimaAutReq_read)
  HmiSecRowRiClimaAutReq_HmiSecRowRiClimaAutReq_IsUpdated/1 -> ClimaCmftStMgr_Runnable_sys/73(HmiSecRowRiClimaAutReq_HmiSecRowRiClimaAutReq_IsUpdated_read)
  ActvnTiForClimaCtrlRe_ActvnTiForClimaCtrlRe/1 -> ClimaCmftStMgr_Runnable_sys/74(ActvnTiForClimaCtrlRe_ActvnTiForClimaCtrlRe_read)
  AirCdnAvlRe_AirCdnAvlRe/1 -> ClimaCmftStMgr_Runnable_sys/75(AirCdnAvlRe_AirCdnAvlRe_read)
  ClimaCfgForBRAND_ClimaCfgForBRAND/1 -> ClimaCmftStMgr_Runnable_sys/76(ClimaCfgForBRAND_ClimaCfgForBRAND_read)
  ClimaCfgForHvacRePrsnt1_ClimaCfgForHvacRePrsnt1/1 -> ClimaCmftStMgr_Runnable_sys/77(ClimaCfgForHvacRePrsnt1_ClimaCfgForHvacRePrsnt1_read)
  ClimaCfgForHvacTyp_ClimaCfgForHvacTyp/1 -> ClimaCmftStMgr_Runnable_sys/78(ClimaCfgForHvacTyp_ClimaCfgForHvacTyp_read)
  ClimaCtrlActvRe_ClimaCtrlActvRe/1 -> ClimaCmftStMgr_Runnable_sys/79(ClimaCtrlActvRe_ClimaCtrlActvRe_read)
  CmptmtAirTEstimdAtRowSec_CmptmtAirTEstimdAtRowSec/1 -> ClimaCmftStMgr_Runnable_sys/80(CmptmtAirTEstimdAtRowSec_CmptmtAirTEstimdAtRowSec_read)
  CmptmtRelHum_Ngcc_CmptmtRelHum/1 -> ClimaCmftStMgr_Runnable_sys/81(CmptmtRelHum_Ngcc_CmptmtRelHum_read)
  CmptmtTFrntRaw_CmptmtTFrntRaw/1 -> ClimaCmftStMgr_Runnable_sys/82(CmptmtTFrntRaw_CmptmtTFrntRaw_read)
  CoupldCtrlErrAtRowSecAvg_CoupldCtrlErrAtRowSecAvg/1 -> ClimaCmftStMgr_Runnable_sys/83(CoupldCtrlErrAtRowSecAvg_CoupldCtrlErrAtRowSecAvg_read)
  EvaprTFrntRaw_EvaprTFrntRaw/1 -> ClimaCmftStMgr_Runnable_sys/84(EvaprTFrntRaw_EvaprTFrntRaw_read)
  HmiAirDistbnRow1LeNgcc_HmiAirDistbnRow1LeNgcc/1 -> ClimaCmftStMgr_Runnable_sys/85(HmiAirDistbnRow1LeNgcc_HmiAirDistbnRow1LeNgcc_read)
  HmiAirDistbnRow1LeNgcc_HmiAirDistbnRow1LeNgcc_IsUpdated/1 -> ClimaCmftStMgr_Runnable_sys/86(HmiAirDistbnRow1LeNgcc_HmiAirDistbnRow1LeNgcc_IsUpdated_read)
  HmiAirDistbnRow1RiNgcc_HmiAirDistbnRow1RiNgcc/1 -> ClimaCmftStMgr_Runnable_sys/87(HmiAirDistbnRow1RiNgcc_HmiAirDistbnRow1RiNgcc_read)
  HmiAirDistbnRow1RiNgcc_HmiAirDistbnRow1RiNgcc_IsUpdated/1 -> ClimaCmftStMgr_Runnable_sys/88(HmiAirDistbnRow1RiNgcc_HmiAirDistbnRow1RiNgcc_IsUpdated_read)
  HmiClimaRow3PwrNgcc_HmiClimaRow3PwrNgcc/1 -> ClimaCmftStMgr_Runnable_sys/89(HmiClimaRow3PwrNgcc_HmiClimaRow3PwrNgcc_read)
  HmiCmptmtAirDistbnFrntLe_HmiCmptmtAirDistbnFrntLe/1 -> ClimaCmftStMgr_Runnable_sys/90(HmiCmptmtAirDistbnFrntLe_HmiCmptmtAirDistbnFrntLe_read)
  HmiCmptmtAirDistbnFrntLe_HmiCmptmtAirDistbnFrntLe_IsUpdated/1 -> ClimaCmftStMgr_Runnable_sys/91(HmiCmptmtAirDistbnFrntLe_HmiCmptmtAirDistbnFrntLe_IsUpdated_read)
  HmiCmptmtAirDistbnFrntRi_HmiCmptmtAirDistbnFrntRi/1 -> ClimaCmftStMgr_Runnable_sys/92(HmiCmptmtAirDistbnFrntRi_HmiCmptmtAirDistbnFrntRi_read)
  HmiCmptmtAirDistbnFrntRi_HmiCmptmtAirDistbnFrntRi_IsUpdated/1 -> ClimaCmftStMgr_Runnable_sys/93(HmiCmptmtAirDistbnFrntRi_HmiCmptmtAirDistbnFrntRi_IsUpdated_read)
  HmiCmptmtAirDistbnRe_HmiCmptmtAirDistbnRe/1 -> ClimaCmftStMgr_Runnable_sys/94(HmiCmptmtAirDistbnRe_HmiCmptmtAirDistbnRe_read)
  HmiCmptmtAirDistbnRe_HmiCmptmtAirDistbnRe_IsUpdated/1 -> ClimaCmftStMgr_Runnable_sys/95(HmiCmptmtAirDistbnRe_HmiCmptmtAirDistbnRe_IsUpdated_read)
  HmiCmptmtCoolgReq_HmiCmptmtCoolgReq/1 -> ClimaCmftStMgr_Runnable_sys/96(HmiCmptmtCoolgReq_HmiCmptmtCoolgReq_read)
  HmiCmptmtCoolgReq_HmiCmptmtCoolgReq_IsUpdated/1 -> ClimaCmftStMgr_Runnable_sys/97(HmiCmptmtCoolgReq_HmiCmptmtCoolgReq_IsUpdated_read)
  HmiCmptmtSecRowLeAirDistbn_HmiCmptmtSecRowLeAirDistbn/1 -> ClimaCmftStMgr_Runnable_sys/98(HmiCmptmtSecRowLeAirDistbn_HmiCmptmtSecRowLeAirDistbn_read)
  HmiCmptmtSecRowLeAirDistbn_HmiCmptmtSecRowLeAirDistbn_IsUpdated/1 -> ClimaCmftStMgr_Runnable_sys/99(HmiCmptmtSecRowLeAirDistbn_HmiCmptmtSecRowLeAirDistbn_IsUpdated_read)
  HmiCmptmtSecRowRiAirDistbn_HmiCmptmtSecRowRiAirDistbn/1 -> ClimaCmftStMgr_Runnable_sys/100(HmiCmptmtSecRowRiAirDistbn_HmiCmptmtSecRowRiAirDistbn_read)
  HmiCmptmtSecRowRiAirDistbn_HmiCmptmtSecRowRiAirDistbn_IsUpdated/1 -> ClimaCmftStMgr_Runnable_sys/101(HmiCmptmtSecRowRiAirDistbn_HmiCmptmtSecRowRiAirDistbn_IsUpdated_read)
  HmiFanLvlRow1LeNgcc_HmiFanLvlRow1LeNgcc/1 -> ClimaCmftStMgr_Runnable_sys/102(HmiFanLvlRow1LeNgcc_HmiFanLvlRow1LeNgcc_read)
  HmiFanLvlRow1LeNgcc_HmiFanLvlRow1LeNgcc_IsUpdated/1 -> ClimaCmftStMgr_Runnable_sys/103(HmiFanLvlRow1LeNgcc_HmiFanLvlRow1LeNgcc_IsUpdated_read)
  HmiFanLvlRow1RiNgcc_HmiFanLvlRow1RiNgcc/1 -> ClimaCmftStMgr_Runnable_sys/104(HmiFanLvlRow1RiNgcc_HmiFanLvlRow1RiNgcc_read)
  HmiFanLvlRow1RiNgcc_HmiFanLvlRow1RiNgcc_IsUpdated/1 -> ClimaCmftStMgr_Runnable_sys/105(HmiFanLvlRow1RiNgcc_HmiFanLvlRow1RiNgcc_IsUpdated_read)
  HmiMaxACReq_HmiMaxACReq/1 -> ClimaCmftStMgr_Runnable_sys/106(HmiMaxACReq_HmiMaxACReq_read)
  HmiMaxACReq_HmiMaxACReq_IsUpdated/1 -> ClimaCmftStMgr_Runnable_sys/107(HmiMaxACReq_HmiMaxACReq_IsUpdated_read)
  HmiPassHvacFanLvl_HmiPassHvacFanLvl/1 -> ClimaCmftStMgr_Runnable_sys/108(HmiPassHvacFanLvl_HmiPassHvacFanLvl_read)
  HmiPassHvacFanLvl_HmiPassHvacFanLvl_IsUpdated/1 -> ClimaCmftStMgr_Runnable_sys/109(HmiPassHvacFanLvl_HmiPassHvacFanLvl_IsUpdated_read)
  HmiSecRowRiHvacFanLvl_HmiSecRowRiHvacFanLvl/1 -> ClimaCmftStMgr_Runnable_sys/110(HmiSecRowRiHvacFanLvl_HmiSecRowRiHvacFanLvl_read)
  HmiSecRowRiHvacFanLvl_HmiSecRowRiHvacFanLvl_IsUpdated/1 -> ClimaCmftStMgr_Runnable_sys/111(HmiSecRowRiHvacFanLvl_HmiSecRowRiHvacFanLvl_IsUpdated_read)
  PrkgClimaACReq_PrkgClimaACReq/1 -> ClimaCmftStMgr_Runnable_sys/112(PrkgClimaACReq_PrkgClimaACReq_read)
  PrkgClimaAirflowReqRe_PrkgClimaAirflowReqRe/1 -> ClimaCmftStMgr_Runnable_sys/113(PrkgClimaAirflowReqRe_PrkgClimaAirflowReqRe_read)
  PrkgClimaOnReqRe_PrkgClimaOnReqRe/1 -> ClimaCmftStMgr_Runnable_sys/114(PrkgClimaOnReqRe_PrkgClimaOnReqRe_read)
  ClimaCmftStMgr_Runnable_sys/1(ClimaStStrtUpRe_ClimaStStrtUpRe_write) -> ClimaStStrtUpRe_ClimaStStrtUpRe/1
  ClimaCmftStMgr_Runnable_sys/2(BiochalPatseldForClima_BiochalPatseldForClima_write) -> BiochalPatseldForClima_BiochalPatseldForClima/1
  ClimaCmftStMgr_Runnable_sys/3(ClimaAtRowFrntActv_ClimaAtRowFrntActv_write) -> ClimaAtRowFrntActv_ClimaAtRowFrntActv/1
  ClimaCmftStMgr_Runnable_sys/4(ClimaAtRowFrntActvLe_ClimaAtRowFrntActvLe_write) -> ClimaAtRowFrntActvLe_ClimaAtRowFrntActvLe/1
  ClimaCmftStMgr_Runnable_sys/5(ClimaAtRowFrntActvRi_ClimaAtRowFrntActvRi_write) -> ClimaAtRowFrntActvRi_ClimaAtRowFrntActvRi/1
  ClimaCmftStMgr_Runnable_sys/6(ClimaAtRowSecActvNgcc_ClimaAtRowSecActv_write) -> ClimaAtRowSecActvNgcc_ClimaAtRowSecActv/1
  ClimaCmftStMgr_Runnable_sys/7(ClimaAtRowSecActvNgcc_ClimaAtRowSecActvLe_write) -> ClimaAtRowSecActvNgcc_ClimaAtRowSecActvLe/1
  ClimaCmftStMgr_Runnable_sys/8(ClimaAtRowSecActvNgcc_ClimaAtRowSecActvRi_write) -> ClimaAtRowSecActvNgcc_ClimaAtRowSecActvRi/1
  ClimaCmftStMgr_Runnable_sys/9(ClimaDefrstMaxReq_ClimaDefrstMaxReq_write) -> ClimaDefrstMaxReq_ClimaDefrstMaxReq/1
  ClimaCmftStMgr_Runnable_sys/10(ClimaReqForFanFrnt_ClimaReqForFanFrnt_write) -> ClimaReqForFanFrnt_ClimaReqForFanFrnt/1
  ClimaCmftStMgr_Runnable_sys/11(ClimaStStrtUp_ClimaStStrtUp_write) -> ClimaStStrtUp_ClimaStStrtUp/1
  ClimaCmftStMgr_Runnable_sys/12(ClimaTSpSpcl_ClimaTSpSpcl_write) -> ClimaTSpSpcl_ClimaTSpSpcl/1
  CmptmtAirTFbExtd_CmptmtAirTFbExtd_merge/1 -> CmptmtAirTFbExtd_CmptmtAirTFbExtd/1
  ClimaCmftStMgr_Runnable_sys/14(CustClimaOvrdNotifForHmiCpt_CustClimaOvrdNotifForHmiCpt_write) -> CustClimaOvrdNotifForHmiCpt_CustClimaOvrdNotifForHmiCpt/1
  ClimaCmftStMgr_Runnable_sys/15(FogFlashPrevnActv_FogFlashPrevnActv_write) -> FogFlashPrevnActv_FogFlashPrevnActv/1
  ClimaCmftStMgr_Runnable_sys/16(HmiCmptmtAirDistbnFrntForClima_HmiCmptmtAirDistbnFrntForClima_write) -> HmiCmptmtAirDistbnFrntForClima_HmiCmptmtAirDistbnFrntForClima/1
  ClimaCmftStMgr_Runnable_sys/17(HmiCmptmtAirDistbnFrntForClima_HmiCmptmtAirDistbnFrntRiForClima_write) -> HmiCmptmtAirDistbnFrntForClima_HmiCmptmtAirDistbnFrntRiForClima/1
  ClimaCmftStMgr_Runnable_sys/18(HmiCmptmtAirDistbnFrntForClima_HmiCmptmtAirDistbnReForClima_write) -> HmiCmptmtAirDistbnFrntForClima_HmiCmptmtAirDistbnReForClima/1
  ClimaCmftStMgr_Runnable_sys/19(HmiCmptmtAirDistbnFrntForClima_HmiCmptmtAirDistbnReRiForClima_write) -> HmiCmptmtAirDistbnFrntForClima_HmiCmptmtAirDistbnReRiForClima/1
  ClimaCmftStMgr_Runnable_sys/20(HmiCmptmtCoolgReqForClima_HmiCmptmtCoolgReqForClima_write) -> HmiCmptmtCoolgReqForClima_HmiCmptmtCoolgReqForClima/1
  HmiCmptmtTSpForClima_HmiCmptmtTSpForClima_merge/1 -> HmiCmptmtTSpForClima_HmiCmptmtTSpForClima/1
  ClimaCmftStMgr_Runnable_sys/22(HmiDefrstMaxReqForClima_HmiDefrstMaxReqForClima_write) -> HmiDefrstMaxReqForClima_HmiDefrstMaxReqForClima/1
  ClimaCmftStMgr_Runnable_sys/23(HmiDrvrClimaAutReqForClima_HmiDrvrClimaAutReqForClima_write) -> HmiDrvrClimaAutReqForClima_HmiDrvrClimaAutReqForClima/1
  ClimaCmftStMgr_Runnable_sys/24(HmiHvacFanLvlFrntForClima_HmiHvacFanLvlFrntForClima_write) -> HmiHvacFanLvlFrntForClima_HmiHvacFanLvlFrntForClima/1
  ClimaCmftStMgr_Runnable_sys/25(HmiHvacFanLvlFrntForClima_HmiHvacFanLvlFrntRiForClima_write) -> HmiHvacFanLvlFrntForClima_HmiHvacFanLvlFrntRiForClima/1
  ClimaCmftStMgr_Runnable_sys/26(HmiHvacFanLvlReForClima_HmiHvacFanLvlReForClima_write) -> HmiHvacFanLvlReForClima_HmiHvacFanLvlReForClima/1
  ClimaCmftStMgr_Runnable_sys/27(HmiHvacFanLvlReForClima_HmiHvacFanLvlReRiForClima_write) -> HmiHvacFanLvlReForClima_HmiHvacFanLvlReRiForClima/1
  ClimaCmftStMgr_Runnable_sys/28(HmiHvacRecircCmdForClima_HmiHvacRecircCmdForClima_write) -> HmiHvacRecircCmdForClima_HmiHvacRecircCmdForClima/1
  ClimaCmftStMgr_Runnable_sys/29(HmiHvacRecircLongPsdStsForClima_HmiHvacRecircLongPsdStsForClima_write) -> HmiHvacRecircLongPsdStsForClima_HmiHvacRecircLongPsdStsForClima/1
  ClimaCmftStMgr_Runnable_sys/30(HmiHvacReCtrlForClima_HmiHvacReCtrlForClima_write) -> HmiHvacReCtrlForClima_HmiHvacReCtrlForClima/1
  ClimaCmftStMgr_Runnable_sys/31(HmiMaxACReqForClima_HmiMaxACReqForClima_write) -> HmiMaxACReqForClima_HmiMaxACReqForClima/1
  ClimaCmftStMgr_Runnable_sys/32(HmiPassClimaAutReqForClima_HmiPassClimaAutReqForClima_write) -> HmiPassClimaAutReqForClima_HmiPassClimaAutReqForClima/1
  ClimaCmftStMgr_Runnable_sys/33(HmiPassTempSyncSetForClima_HmiPassTempSyncSetForClima_write) -> HmiPassTempSyncSetForClima_HmiPassTempSyncSetForClima/1
  ClimaCmftStMgr_Runnable_sys/34(HmiPopUpRespForClima_HmiPopUpRespForClima_write) -> HmiPopUpRespForClima_HmiPopUpRespForClima/1
  ClimaCmftStMgr_Runnable_sys/35(HmiSecRowLeClimaAutReqForClima_HmiSecRowLeClimaAutReqForClima_write) -> HmiSecRowLeClimaAutReqForClima_HmiSecRowLeClimaAutReqForClima/1
  ClimaCmftStMgr_Runnable_sys/36(HmiSecRowRiClimaAutReqForClima_HmiSecRowRiClimaAutReqForClima_write) -> HmiSecRowRiClimaAutReqForClima_HmiSecRowRiClimaAutReqForClima/1
  ClimaCmftStMgr_Runnable_sys/37(MemSaveFlgForHmi_MemSaveFlgForHmi_write) -> MemSaveFlgForHmi_MemSaveFlgForHmi/1
  MemWrForHmi_MemWrForHmi_merge/1 -> MemWrForHmi_MemWrForHmi/1
  ClimaCmftStMgr_Runnable_sys/39(VFCHmiClimaCtrlAct_VFCHmiClimaCtrlAct_write) -> VFCHmiClimaCtrlAct_VFCHmiClimaCtrlAct/1
  ClimaCmftStMgr_Runnable_sys/38(MemWrForHmi_MemWrForHmi_write) -> [MemWrForHmi_MemWrForHmi_merge_Tag]
  [MemWrForHmi_MemWrForHmi_merge_Tag] -> MemWrForHmi_MemWrForHmi_merge/2
  ClimaCmftStMgr_Init/3(MemWrForHmi_MemWrForHmi_write) -> [MemWrForHmi_MemWrForHmi_merge_Tag1]
  [MemWrForHmi_MemWrForHmi_merge_Tag1] -> MemWrForHmi_MemWrForHmi_merge/1
  ClimaCmftStMgr_Runnable_sys/21(HmiCmptmtTSpForClima_HmiCmptmtTSpForClima_write) -> [HmiCmptmtTSpForClima_HmiCmptmtTSpForClima_merge_Tag]
  [HmiCmptmtTSpForClima_HmiCmptmtTSpForClima_merge_Tag] -> HmiCmptmtTSpForClima_HmiCmptmtTSpForClima_merge/2
  ClimaCmftStMgr_Init/2(HmiCmptmtTSpForClima_HmiCmptmtTSpForClima_write) -> [HmiCmptmtTSpForClima_HmiCmptmtTSpForClima_merge_Tag1]
  [HmiCmptmtTSpForClima_HmiCmptmtTSpForClima_merge_Tag1] -> HmiCmptmtTSpForClima_HmiCmptmtTSpForClima_merge/1
  ClimaCmftStMgr_Runnable_sys/13(CmptmtAirTFbExtd_CmptmtAirTFbExtd_write) -> [CmptmtAirTFbExtd_CmptmtAirTFbExtd_merge_Tag]
  [CmptmtAirTFbExtd_CmptmtAirTFbExtd_merge_Tag] -> CmptmtAirTFbExtd_CmptmtAirTFbExtd_merge/2
  ClimaCmftStMgr_Init/1(CmptmtAirTFbExtd_CmptmtAirTFbExtd_write) -> [CmptmtAirTFbExtd_CmptmtAirTFbExtd_merge_Tag1]
  [CmptmtAirTFbExtd_CmptmtAirTFbExtd_merge_Tag1] -> CmptmtAirTFbExtd_CmptmtAirTFbExtd_merge/1
  ClimaCmftStMgr_Runnable/1 -> ClimaCmftStMgr_Runnable_sys/Trigger

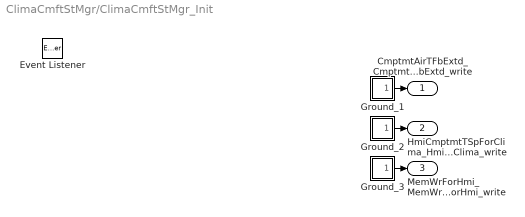
== system ClimaCmftStMgr/ClimaCmftStMgr_Init ==
  Event Listener : EventListener  EventName=reset
  Ground_1 : Subsystem
  Ground_2 : Subsystem
  Ground_3 : Subsystem
  CmptmtAirTFbExtd_CmptmtAirTFbExtd_write : Outport
  HmiCmptmtTSpForClima_HmiCmptmtTSpForClima_write : Outport  Port=2
  MemWrForHmi_MemWrForHmi_write : Outport  Port=3
  wires:
  Ground_1/1(Out1) -> CmptmtAirTFbExtd_CmptmtAirTFbExtd_write/1
  Ground_2/1(Out1) -> HmiCmptmtTSpForClima_HmiCmptmtTSpForClima_write/1
  Ground_3/1(Out1) -> MemWrForHmi_MemWrForHmi_write/1
